simulink model "dds_cwg_32k_dual_test"  (R2016b) (continued)
== system dds_cwg_32k_dual_test/DDC == (continued)
      | pipeline_init(gcb,'latency',latency);
      settings: AttributesFormatString=Latency = %<latency>
      mask Display:
      | port_label('input', 1, 'd');
      | port_label('output', 1, 'q');
      | disp(['Z^-', num2str(latency)]);
  pipeline11 : Subsystem  Commented=on  AncestorBlock=casper_library_delays/pipeline  mask(pipeline){latency=2}
      mask help:
      | An explicitly laid-out delay line to avoid mapping to SRL16s
      | for use in pipelining to help achieve timing closure.
      mask init:
      | pipeline_init(gcb,'latency',latency);
      settings: AttributesFormatString=Latency = %<latency>
      mask Display:
      | port_label('input', 1, 'd');
      | port_label('output', 1, 'q');
      | disp(['Z^-', num2str(latency)]);
  pipeline12 : Subsystem  Commented=on  AncestorBlock=casper_library_delays/pipeline  mask(pipeline){latency=2}
      mask help:
      | An explicitly laid-out delay line to avoid mapping to SRL16s
      | for use in pipelining to help achieve timing closure.
      mask init:
      | pipeline_init(gcb,'latency',latency);
      settings: AttributesFormatString=Latency = %<latency>
      mask Display:
      | port_label('input', 1, 'd');
      | port_label('output', 1, 'q');
      | disp(['Z^-', num2str(latency)]);
  pipeline13 : Subsystem  Commented=on  AncestorBlock=casper_library_delays/pipeline  mask(pipeline){latency=2}
      mask help:
      | An explicitly laid-out delay line to avoid mapping to SRL16s
      | for use in pipelining to help achieve timing closure.
      mask init:
      | pipeline_init(gcb,'latency',latency);
      settings: AttributesFormatString=Latency = %<latency>
      mask Display:
      | port_label('input', 1, 'd');
      | port_label('output', 1, 'q');
      | disp(['Z^-', num2str(latency)]);
  pipeline14 : Subsystem  Commented=on  AncestorBlock=casper_library_delays/pipeline  mask(pipeline){latency=2}
      mask help:
      | An explicitly laid-out delay line to avoid mapping to SRL16s
      | for use in pipelining to help achieve timing closure.
      mask init:
      | pipeline_init(gcb,'latency',latency);
      settings: AttributesFormatString=Latency = %<latency>
      mask Display:
      | port_label('input', 1, 'd');
      | port_label('output', 1, 'q');
      | disp(['Z^-', num2str(latency)]);
  pipeline15 : Subsystem  Commented=on  AncestorBlock=casper_library_delays/pipeline  mask(pipeline){latency=2}
      mask help:
      | An explicitly laid-out delay line to avoid mapping to SRL16s
      | for use in pipelining to help achieve timing closure.
      mask init:
      | pipeline_init(gcb,'latency',latency);
      settings: AttributesFormatString=Latency = %<latency>
      mask Display:
      | port_label('input', 1, 'd');
      | port_label('output', 1, 'q');
      | disp(['Z^-', num2str(latency)]);
  pipeline16 : Subsystem  Commented=on  AncestorBlock=casper_library_delays/pipeline  mask(pipeline){latency=2}
      mask help:
      | An explicitly laid-out delay line to avoid mapping to SRL16s
      | for use in pipelining to help achieve timing closure.
      mask init:
      | pipeline_init(gcb,'latency',latency);
      settings: AttributesFormatString=Latency = %<latency>
      mask Display:
      | port_label('input', 1, 'd');
      | port_label('output', 1, 'q');
      | disp(['Z^-', num2str(latency)]);
  pipeline17 : Subsystem  Commented=on  AncestorBlock=casper_library_delays/pipeline  mask(pipeline){latency=2}
      mask help:
      | An explicitly laid-out delay line to avoid mapping to SRL16s
      | for use in pipelining to help achieve timing closure.
      mask init:
      | pipeline_init(gcb,'latency',latency);
      settings: AttributesFormatString=Latency = %<latency>
      mask Display:
      | port_label('input', 1, 'd');
      | port_label('output', 1, 'q');
      | disp(['Z^-', num2str(latency)]);
  pipeline18 : Subsystem  Commented=on  AncestorBlock=casper_library_delays/pipeline  mask(pipeline){latency=2}
      mask help:
      | An explicitly laid-out delay line to avoid mapping to SRL16s
      | for use in pipelining to help achieve timing closure.
      mask init:
      | pipeline_init(gcb,'latency',latency);
      settings: AttributesFormatString=Latency = %<latency>
      mask Display:
      | port_label('input', 1, 'd');
      | port_label('output', 1, 'q');
      | disp(['Z^-', num2str(latency)]);
  pipeline19 : Subsystem  Commented=on  AncestorBlock=casper_library_delays/pipeline  mask(pipeline){latency=2}
      mask help:
      | An explicitly laid-out delay line to avoid mapping to SRL16s
      | for use in pipelining to help achieve timing closure.
      mask init:
      | pipeline_init(gcb,'latency',latency);
      settings: AttributesFormatString=Latency = %<latency>
      mask Display:
      | port_label('input', 1, 'd');
      | port_label('output', 1, 'q');
      | disp(['Z^-', num2str(latency)]);
  pipeline2 : Subsystem  Commented=on  AncestorBlock=casper_library_delays/pipeline  mask(pipeline){latency=1}
      mask help:
      | An explicitly laid-out delay line to avoid mapping to SRL16s
      | for use in pipelining to help achieve timing closure.
      mask init:
      | pipeline_init(gcb,'latency',latency);
      settings: AttributesFormatString=Latency = %<latency>
      mask Display:
      | port_label('input', 1, 'd');
      | port_label('output', 1, 'q');
      | disp(['Z^-', num2str(latency)]);
  pipeline20 : Subsystem  Commented=on  AncestorBlock=casper_library_delays/pipeline  mask(pipeline){latency=2}
      mask help:
      | An explicitly laid-out delay line to avoid mapping to SRL16s
      | for use in pipelining to help achieve timing closure.
      mask init:
      | pipeline_init(gcb,'latency',latency);
      settings: AttributesFormatString=Latency = %<latency>
      mask Display:
      | port_label('input', 1, 'd');
      | port_label('output', 1, 'q');
      | disp(['Z^-', num2str(latency)]);
  pipeline21 : Subsystem  AncestorBlock=casper_library_delays/pipeline  mask(pipeline){latency=2}
      mask help:
      | An explicitly laid-out delay line to avoid mapping to SRL16s
      | for use in pipelining to help achieve timing closure.
      mask init:
      | pipeline_init(gcb,'latency',latency);
      settings: AttributesFormatString=Latency = %<latency>
      mask Display:
      | port_label('input', 1, 'd');
      | port_label('output', 1, 'q');
      | disp(['Z^-', num2str(latency)]);
  pipeline22 : Subsystem  AncestorBlock=casper_library_delays/pipeline  mask(pipeline){latency=2}
      mask help:
      | An explicitly laid-out delay line to avoid mapping to SRL16s
      | for use in pipelining to help achieve timing closure.
      mask init:
      | pipeline_init(gcb,'latency',latency);
      settings: AttributesFormatString=Latency = %<latency>
      mask Display:
      | port_label('input', 1, 'd');
      | port_label('output', 1, 'q');
      | disp(['Z^-', num2str(latency)]);
  pipeline23 : Subsystem  Commented=on  AncestorBlock=casper_library_delays/pipeline  mask(pipeline){latency=2}
      mask help:
      | An explicitly laid-out delay line to avoid mapping to SRL16s
      | for use in pipelining to help achieve timing closure.
      mask init:
      | pipeline_init(gcb,'latency',latency);
      settings: AttributesFormatString=Latency = %<latency>
      mask Display:
      | port_label('input', 1, 'd');
      | port_label('output', 1, 'q');
      | disp(['Z^-', num2str(latency)]);
  pipeline24 : Subsystem  Commented=on  AncestorBlock=casper_library_delays/pipeline  mask(pipeline){latency=2}
      mask help:
      | An explicitly laid-out delay line to avoid mapping to SRL16s
      | for use in pipelining to help achieve timing closure.
      mask init:
      | pipeline_init(gcb,'latency',latency);
      settings: AttributesFormatString=Latency = %<latency>
      mask Display:
      | port_label('input', 1, 'd');
      | port_label('output', 1, 'q');
      | disp(['Z^-', num2str(latency)]);
  pipeline25 : Subsystem  Commented=on  AncestorBlock=casper_library_delays/pipeline  mask(pipeline){latency=1}
      mask help:
      | An explicitly laid-out delay line to avoid mapping to SRL16s
      | for use in pipelining to help achieve timing closure.
      mask init:
      | pipeline_init(gcb,'latency',latency);
      settings: AttributesFormatString=Latency = %<latency>
      mask Display:
      | port_label('input', 1, 'd');
      | port_label('output', 1, 'q');
      | disp(['Z^-', num2str(latency)]);
  pipeline26 : Subsystem  Commented=on  AncestorBlock=casper_library_delays/pipeline  mask(pipeline){latency=2}
      mask help:
      | An explicitly laid-out delay line to avoid mapping to SRL16s
      | for use in pipelining to help achieve timing closure.
      mask init:
      | pipeline_init(gcb,'latency',latency);
      settings: AttributesFormatString=Latency = %<latency>
      mask Display:
      | port_label('input', 1, 'd');
      | port_label('output', 1, 'q');
      | disp(['Z^-', num2str(latency)]);
  pipeline27 : Subsystem  Commented=on  AncestorBlock=casper_library_delays/pipeline  mask(pipeline){latency=2}
      mask help:
      | An explicitly laid-out delay line to avoid mapping to SRL16s
      | for use in pipelining to help achieve timing closure.
      mask init:
      | pipeline_init(gcb,'latency',latency);
      settings: AttributesFormatString=Latency = %<latency>
      mask Display:
      | port_label('input', 1, 'd');
      | port_label('output', 1, 'q');
      | disp(['Z^-', num2str(latency)]);
  pipeline28 : Subsystem  Commented=on  AncestorBlock=casper_library_delays/pipeline  mask(pipeline){latency=2}
      mask help:
      | An explicitly laid-out delay line to avoid mapping to SRL16s
      | for use in pipelining to help achieve timing closure.
      mask init:
      | pipeline_init(gcb,'latency',latency);
      settings: AttributesFormatString=Latency = %<latency>
      mask Display:
      | port_label('input', 1, 'd');
      | port_label('output', 1, 'q');
      | disp(['Z^-', num2str(latency)]);
  pipeline29 : Subsystem  Commented=on  AncestorBlock=casper_library_delays/pipeline  mask(pipeline){latency=2}
      mask help:
      | An explicitly laid-out delay line to avoid mapping to SRL16s
      | for use in pipelining to help achieve timing closure.
      mask init:
      | pipeline_init(gcb,'latency',latency);
      settings: AttributesFormatString=Latency = %<latency>
      mask Display:
      | port_label('input', 1, 'd');
      | port_label('output', 1, 'q');
      | disp(['Z^-', num2str(latency)]);
  pipeline3 : Subsystem  Commented=on  AncestorBlock=casper_library_delays/pipeline  mask(pipeline){latency=2}
      mask help:
      | An explicitly laid-out delay line to avoid mapping to SRL16s
      | for use in pipelining to help achieve timing closure.
      mask init:
      | pipeline_init(gcb,'latency',latency);
      settings: AttributesFormatString=Latency = %<latency>
      mask Display:
      | port_label('input', 1, 'd');
      | port_label('output', 1, 'q');
      | disp(['Z^-', num2str(latency)]);
  pipeline30 : Subsystem  Commented=on  AncestorBlock=casper_library_delays/pipeline  mask(pipeline){latency=2}
      mask help:
      | An explicitly laid-out delay line to avoid mapping to SRL16s
      | for use in pipelining to help achieve timing closure.
      mask init:
      | pipeline_init(gcb,'latency',latency);
      settings: AttributesFormatString=Latency = %<latency>
      mask Display:
      | port_label('input', 1, 'd');
      | port_label('output', 1, 'q');
      | disp(['Z^-', num2str(latency)]);
  pipeline31 : Subsystem  Commented=on  AncestorBlock=casper_library_delays/pipeline  mask(pipeline){latency=2}
      mask help:
      | An explicitly laid-out delay line to avoid mapping to SRL16s
      | for use in pipelining to help achieve timing closure.
      mask init:
      | pipeline_init(gcb,'latency',latency);
      settings: AttributesFormatString=Latency = %<latency>
      mask Display:
      | port_label('input', 1, 'd');
      | port_label('output', 1, 'q');
      | disp(['Z^-', num2str(latency)]);
  pipeline32 : Subsystem  Commented=on  AncestorBlock=casper_library_delays/pipeline  mask(pipeline){latency=1}
      mask help:
      | An explicitly laid-out delay line to avoid mapping to SRL16s
      | for use in pipelining to help achieve timing closure.
      mask init:
      | pipeline_init(gcb,'latency',latency);
      settings: AttributesFormatString=Latency = %<latency>
      mask Display:
      | port_label('input', 1, 'd');
      | port_label('output', 1, 'q');
      | disp(['Z^-', num2str(latency)]);
  pipeline33 : Subsystem  Commented=on  AncestorBlock=casper_library_delays/pipeline  mask(pipeline){latency=2}
      mask help:
      | An explicitly laid-out delay line to avoid mapping to SRL16s
      | for use in pipelining to help achieve timing closure.
      mask init:
      | pipeline_init(gcb,'latency',latency);
      settings: AttributesFormatString=Latency = %<latency>
      mask Display:
      | port_label('input', 1, 'd');
      | port_label('output', 1, 'q');
      | disp(['Z^-', num2str(latency)]);
  pipeline34 : Subsystem  Commented=on  AncestorBlock=casper_library_delays/pipeline  mask(pipeline){latency=2}
      mask help:
      | An explicitly laid-out delay line to avoid mapping to SRL16s
      | for use in pipelining to help achieve timing closure.
      mask init:
      | pipeline_init(gcb,'latency',latency);
      settings: AttributesFormatString=Latency = %<latency>
      mask Display:
      | port_label('input', 1, 'd');
      | port_label('output', 1, 'q');
      | disp(['Z^-', num2str(latency)]);
  pipeline35 : Subsystem  Commented=on  AncestorBlock=casper_library_delays/pipeline  mask(pipeline){latency=2}
      mask help:
      | An explicitly laid-out delay line to avoid mapping to SRL16s
      | for use in pipelining to help achieve timing closure.
      mask init:
      | pipeline_init(gcb,'latency',latency);
      settings: AttributesFormatString=Latency = %<latency>
      mask Display:
      | port_label('input', 1, 'd');
      | port_label('output', 1, 'q');
      | disp(['Z^-', num2str(latency)]);
  pipeline36 : Subsystem  Commented=on  AncestorBlock=casper_library_delays/pipeline  mask(pipeline){latency=2}
      mask help:
      | An explicitly laid-out delay line to avoid mapping to SRL16s
      | for use in pipelining to help achieve timing closure.
      mask init:
      | pipeline_init(gcb,'latency',latency);
      settings: AttributesFormatString=Latency = %<latency>
      mask Display:
      | port_label('input', 1, 'd');
      | port_label('output', 1, 'q');
      | disp(['Z^-', num2str(latency)]);
  pipeline37 : Subsystem  Commented=on  AncestorBlock=casper_library_delays/pipeline  mask(pipeline){latency=2}
      mask help:
      | An explicitly laid-out delay line to avoid mapping to SRL16s
      | for use in pipelining to help achieve timing closure.
      mask init:
      | pipeline_init(gcb,'latency',latency);
      settings: AttributesFormatString=Latency = %<latency>
      mask Display:
      | port_label('input', 1, 'd');
      | port_label('output', 1, 'q');
      | disp(['Z^-', num2str(latency)]);
  pipeline38 : Subsystem  Commented=on  AncestorBlock=casper_library_delays/pipeline  mask(pipeline){latency=2}
      mask help:
      | An explicitly laid-out delay line to avoid mapping to SRL16s
      | for use in pipelining to help achieve timing closure.
      mask init:
      | pipeline_init(gcb,'latency',latency);
      settings: AttributesFormatString=Latency = %<latency>
      mask Display:
      | port_label('input', 1, 'd');
      | port_label('output', 1, 'q');
      | disp(['Z^-', num2str(latency)]);
  pipeline39 : Subsystem  AncestorBlock=casper_library_delays/pipeline  mask(pipeline){latency=2}
      mask help:
      | An explicitly laid-out delay line to avoid mapping to SRL16s
      | for use in pipelining to help achieve timing closure.
      mask init:
      | pipeline_init(gcb,'latency',latency);
      settings: AttributesFormatString=Latency = %<latency>
      mask Display:
      | port_label('input', 1, 'd');
      | port_label('output', 1, 'q');
      | disp(['Z^-', num2str(latency)]);
  pipeline4 : Subsystem  Commented=on  AncestorBlock=casper_library_delays/pipeline  mask(pipeline){latency=2}
      mask help:
      | An explicitly laid-out delay line to avoid mapping to SRL16s
      | for use in pipelining to help achieve timing closure.
      mask init:
      | pipeline_init(gcb,'latency',latency);
      settings: AttributesFormatString=Latency = %<latency>
      mask Display:
      | port_label('input', 1, 'd');
      | port_label('output', 1, 'q');
      | disp(['Z^-', num2str(latency)]);
  pipeline40 : Subsystem  AncestorBlock=casper_library_delays/pipeline  mask(pipeline){latency=2}
      mask help:
      | An explicitly laid-out delay line to avoid mapping to SRL16s
      | for use in pipelining to help achieve timing closure.
      mask init:
      | pipeline_init(gcb,'latency',latency);
      settings: AttributesFormatString=Latency = %<latency>
      mask Display:
      | port_label('input', 1, 'd');
      | port_label('output', 1, 'q');
      | disp(['Z^-', num2str(latency)]);
  pipeline41 : Subsystem  Commented=on  AncestorBlock=casper_library_delays/pipeline  mask(pipeline){latency=2}
      mask help:
      | An explicitly laid-out delay line to avoid mapping to SRL16s
      | for use in pipelining to help achieve timing closure.
      mask init:
      | pipeline_init(gcb,'latency',latency);
      settings: AttributesFormatString=Latency = %<latency>
      mask Display:
      | port_label('input', 1, 'd');
      | port_label('output', 1, 'q');
      | disp(['Z^-', num2str(latency)]);
  pipeline42 : Subsystem  Commented=on  AncestorBlock=casper_library_delays/pipeline  mask(pipeline){latency=2}
      mask help:
      | An explicitly laid-out delay line to avoid mapping to SRL16s
      | for use in pipelining to help achieve timing closure.
      mask init:
      | pipeline_init(gcb,'latency',latency);
      settings: AttributesFormatString=Latency = %<latency>
      mask Display:
      | port_label('input', 1, 'd');
      | port_label('output', 1, 'q');
      | disp(['Z^-', num2str(latency)]);
  pipeline43 : Subsystem  Commented=on  AncestorBlock=casper_library_delays/pipeline  mask(pipeline){latency=2}
      mask help:
      | An explicitly laid-out delay line to avoid mapping to SRL16s
      | for use in pipelining to help achieve timing closure.
      mask init:
      | pipeline_init(gcb,'latency',latency);
      settings: AttributesFormatString=Latency = %<latency>
      mask Display:
      | port_label('input', 1, 'd');
      | port_label('output', 1, 'q');
      | disp(['Z^-', num2str(latency)]);
  pipeline44 : Subsystem  Commented=on  AncestorBlock=casper_library_delays/pipeline  mask(pipeline){latency=1}
      mask help:
      | An explicitly laid-out delay line to avoid mapping to SRL16s
      | for use in pipelining to help achieve timing closure.
      mask init:
      | pipeline_init(gcb,'latency',latency);
      settings: AttributesFormatString=Latency = %<latency>
      mask Display:
      | port_label('input', 1, 'd');
      | port_label('output', 1, 'q');
      | disp(['Z^-', num2str(latency)]);
  pipeline5 : Subsystem  Commented=on  AncestorBlock=casper_library_delays/pipeline  mask(pipeline){latency=2}
      mask help:
      | An explicitly laid-out delay line to avoid mapping to SRL16s
      | for use in pipelining to help achieve timing closure.
      mask init:
      | pipeline_init(gcb,'latency',latency);
      settings: AttributesFormatString=Latency = %<latency>
      mask Display:
      | port_label('input', 1, 'd');
      | port_label('output', 1, 'q');
      | disp(['Z^-', num2str(latency)]);
  pipeline6 : Subsystem  Commented=on  AncestorBlock=casper_library_delays/pipeline  mask(pipeline){latency=2}
      mask help:
      | An explicitly laid-out delay line to avoid mapping to SRL16s
      | for use in pipelining to help achieve timing closure.
      mask init:
      | pipeline_init(gcb,'latency',latency);
      settings: AttributesFormatString=Latency = %<latency>
      mask Display:
      | port_label('input', 1, 'd');
      | port_label('output', 1, 'q');
      | disp(['Z^-', num2str(latency)]);
  pipeline7 : Subsystem  Commented=on  AncestorBlock=casper_library_delays/pipeline  mask(pipeline){latency=2}
      mask help:
      | An explicitly laid-out delay line to avoid mapping to SRL16s
      | for use in pipelining to help achieve timing closure.
      mask init:
      | pipeline_init(gcb,'latency',latency);
      settings: AttributesFormatString=Latency = %<latency>
      mask Display:
      | port_label('input', 1, 'd');
      | port_label('output', 1, 'q');
      | disp(['Z^-', num2str(latency)]);
  pipeline8 : Subsystem  Commented=on  AncestorBlock=casper_library_delays/pipeline  mask(pipeline){latency=2}
      mask help:
      | An explicitly laid-out delay line to avoid mapping to SRL16s
      | for use in pipelining to help achieve timing closure.
      mask init:
      | pipeline_init(gcb,'latency',latency);
      settings: AttributesFormatString=Latency = %<latency>
      mask Display:
      | port_label('input', 1, 'd');
      | port_label('output', 1, 'q');
      | disp(['Z^-', num2str(latency)]);
  pipeline9 : Subsystem  Commented=on  AncestorBlock=casper_library_delays/pipeline  mask(pipeline){latency=2}
      mask help:
      | An explicitly laid-out delay line to avoid mapping to SRL16s
      | for use in pipelining to help achieve timing closure.
      mask init:
      | pipeline_init(gcb,'latency',latency);
      settings: AttributesFormatString=Latency = %<latency>
      mask Display:
      | port_label('input', 1, 'd');
      | port_label('output', 1, 'q');
      | disp(['Z^-', num2str(latency)]);
  ri_to_c1 : casper_library_misc/ri_to_c  Commented=on
  snap_ddc : Subsystem  Tag=casper:bitsnap  Commented=on  AncestorBlock=casper_library_scopes/bitfield_snapshot  mask(bitsnap){io_names=[fir_re fir_re_u fir_im dv sync], io_widths=[18 18 18 1 1], io_bps=[17 0 17 0 0], io_types=[1 0 1 2 2], show_format=on, snap_storage=bram, snap_dram_dimm=2, snap_dram_clock=250, snap_nsamples=10, snap_data_width=64, snap_offset=on, snap_circap=off, snap_value=off, snap_ext_arm=off, snap_ext_circ=off, snap_provide_outputs=on, snap_use_dsp48=on, snap_delay=0, extra_names=[c d], extra_widths=[30 2], extra_bps=[0 0], extra_types=0}
      mask prompts: io_names "Names [msb...lsb]"; io_widths "Bitwidths [msb...lsb]"; io_bps "Binary pts [msb...lsb]"; io_types "Data types [msb...lsb], ufix=0, fix=1, bool=2"; show_format "Print format string?"; snap_storage "Storage medium"; snap_dram_dimm "DRAM dimm"; snap_dram_clock "DRAM clock rate"; snap_nsamples "Number of Samples (2^?)"; snap_data_width "Data width"; snap_offset "Start delay support"; snap_circap "Circular capture support"; snap_value "Extra value capture support"; snap_ext_arm "External arm support"; snap_ext_circ "External circular capture support"; snap_provide_outputs "Provide outputs"; snap_use_dsp48 "Use DSP48s to implement counters"; snap_delay "Pre-snapshot delay"; extra_names "Names [msb...lsb]"; extra_widths "Widths [msb...lsb]"; extra_bps "Binary pts [msb...lsb]"; extra_types "Data types [msb...lsb], ufix=0, fix=1, bool=2"
      mask help:
      | A wrapped snapshot block. Allows specification of inputs to a snapblock word so the toolflow can automatically set up the scope.
      | See documentation for snapshot for explanation of parameters.
      mask init:
      | bitsnap_init(gcb);
      settings: AttributesFormatString=|
      | snap(5):f18.17,uf18.0,f18.17,b,b = 56 bits\n64 wide, 1024 deep
      | debugID: 41605630
      mask TypeOptions snap_storage: bram | dram
      mask Callback snap_storage: bitsnap_callback();
      mask TypeOptions snap_dram_dimm: 1 | 2 | 3 | 4
      mask TypeOptions snap_dram_clock: 200 | 250 | 266 | 300 | 333
      mask TypeOptions snap_data_width: 8 | 16 | 32 | 64 | 128
      mask Callback snap_value: bitsnap_callback();
      mask Callback snap_ext_arm: bitsnap_callback();
      mask Callback snap_ext_circ: bitsnap_callback();
      mask Callback snap_provide_outputs: bitsnap_callback();
  snap_ddc_dgb : Subsystem  Tag=casper:bitsnap  Commented=on  AncestorBlock=casper_library_scopes/bitfield_snapshot  mask(bitsnap){io_names=[dec_r1 dec_i1 dec_r9 dec_i9 sync_in dv_in ddc_rout ddc_iout sync_out dv_out], io_widths=[18 18 18 18 1 1 18 18 1 1], io_bps=[17 17 17 17 0 0 17 17 0 0], io_types=[1 1 1 1 2 2 1 1 2 2], show_format=on, snap_storage=bram, snap_dram_dimm=2, snap_dram_clock=250, snap_nsamples=10, snap_data_width=128, snap_offset=on, snap_circap=off, snap_value=off, snap_ext_arm=off, snap_ext_circ=off, snap_provide_outputs=on, snap_use_dsp48=on, snap_delay=0, extra_names=[c d], extra_widths=[30 2], extra_bps=[0 0], extra_types=0}
      mask prompts: io_names "Names [msb...lsb]"; io_widths "Bitwidths [msb...lsb]"; io_bps "Binary pts [msb...lsb]"; io_types "Data types [msb...lsb], ufix=0, fix=1, bool=2"; show_format "Print format string?"; snap_storage "Storage medium"; snap_dram_dimm "DRAM dimm"; snap_dram_clock "DRAM clock rate"; snap_nsamples "Number of Samples (2^?)"; snap_data_width "Data width"; snap_offset "Start delay support"; snap_circap "Circular capture support"; snap_value "Extra value capture support"; snap_ext_arm "External arm support"; snap_ext_circ "External circular capture support"; snap_provide_outputs "Provide outputs"; snap_use_dsp48 "Use DSP48s to implement counters"; snap_delay "Pre-snapshot delay"; extra_names "Names [msb...lsb]"; extra_widths "Widths [msb...lsb]"; extra_bps "Binary pts [msb...lsb]"; extra_types "Data types [msb...lsb], ufix=0, fix=1, bool=2"
      mask help:
      | A wrapped snapshot block. Allows specification of inputs to a snapblock word so the toolflow can automatically set up the scope.
      | See documentation for snapshot for explanation of parameters.
      mask init:
      | bitsnap_init(gcb);
      settings: AttributesFormatString=|
      | snap(10):f18.17,f18.17,f18.17,f18.17,b,b,f18.17,f18.17,b,b = 112 bits\n128 wide, 1024 deep
      | debugID: 108545790
      mask TypeOptions snap_storage: bram | dram
      mask Callback snap_storage: bitsnap_callback();
      mask TypeOptions snap_dram_dimm: 1 | 2 | 3 | 4
      mask TypeOptions snap_dram_clock: 200 | 250 | 266 | 300 | 333
      mask TypeOptions snap_data_width: 8 | 16 | 32 | 64 | 128
      mask Callback snap_value: bitsnap_callback();
      mask Callback snap_ext_arm: bitsnap_callback();
      mask Callback snap_ext_circ: bitsnap_callback();
      mask Callback snap_provide_outputs: bitsnap_callback();
  snap_mix : Subsystem  Tag=casper:bitsnap  Commented=on  AncestorBlock=casper_library_scopes/bitfield_snapshot  mask(bitsnap){io_names=[mix0 mix7 sync dv], io_widths=[18 18 1 1], io_bps=[17 0 0 0], io_types=[1 0 2 2], show_format=on, snap_storage=bram, snap_dram_dimm=2, snap_dram_clock=250, snap_nsamples=10, snap_data_width=64, snap_offset=on, snap_circap=off, snap_value=off, snap_ext_arm=off, snap_ext_circ=off, snap_provide_outputs=on, snap_use_dsp48=on, snap_delay=0, extra_names=[c d], extra_widths=[30 2], extra_bps=[0 0], extra_types=0}
      mask prompts: io_names "Names [msb...lsb]"; io_widths "Bitwidths [msb...lsb]"; io_bps "Binary pts [msb...lsb]"; io_types "Data types [msb...lsb], ufix=0, fix=1, bool=2"; show_format "Print format string?"; snap_storage "Storage medium"; snap_dram_dimm "DRAM dimm"; snap_dram_clock "DRAM clock rate"; snap_nsamples "Number of Samples (2^?)"; snap_data_width "Data width"; snap_offset "Start delay support"; snap_circap "Circular capture support"; snap_value "Extra value capture support"; snap_ext_arm "External arm support"; snap_ext_circ "External circular capture support"; snap_provide_outputs "Provide outputs"; snap_use_dsp48 "Use DSP48s to implement counters"; snap_delay "Pre-snapshot delay"; extra_names "Names [msb...lsb]"; extra_widths "Widths [msb...lsb]"; extra_bps "Binary pts [msb...lsb]"; extra_types "Data types [msb...lsb], ufix=0, fix=1, bool=2"
      mask help:
      | A wrapped snapshot block. Allows specification of inputs to a snapblock word so the toolflow can automatically set up the scope.
      | See documentation for snapshot for explanation of parameters.
      mask init:
      | bitsnap_init(gcb);
      settings: AttributesFormatString=|
      | snap(4):f18.17,uf18.0,b,b = 38 bits\n64 wide, 1024 deep
      | debugID: 36075749
      mask TypeOptions snap_storage: bram | dram
      mask Callback snap_storage: bitsnap_callback();
      mask TypeOptions snap_dram_dimm: 1 | 2 | 3 | 4
      mask TypeOptions snap_dram_clock: 200 | 250 | 266 | 300 | 333
      mask TypeOptions snap_data_width: 8 | 16 | 32 | 64 | 128
      mask Callback snap_value: bitsnap_callback();
      mask Callback snap_ext_arm: bitsnap_callback();
      mask Callback snap_ext_circ: bitsnap_callback();
      mask Callback snap_provide_outputs: bitsnap_callback();
  snap_mult : Subsystem  Tag=casper:bitsnap  Commented=on  AncestorBlock=casper_library_scopes/bitfield_snapshot  mask(bitsnap){io_names=[ain0 ain7 bin0 bin7 mout0 mix0 mix7 sync_in dv_in mix_sync mix_dv dec_sync dec_dv], io_widths=[10 10 18 18 18 18 18 1 1 1 1 1 1], io_bps=[9 0 17 0 17 17 0 0 0 0 0 0 0], io_types=[1 0 1 0 1 1 0 2 2 2 2 2 2], show_format=on, snap_storage=bram, snap_dram_dimm=2, snap_dram_clock=250, snap_nsamples=10, snap_data_width=128, snap_offset=on, snap_circap=off, snap_value=off, snap_ext_arm=off, snap_ext_circ=off, snap_provide_outputs=on, snap_use_dsp48=on, snap_delay=0, extra_names=[c d], extra_widths=[30 2], extra_bps=[0 0], extra_types=0}
      mask prompts: io_names "Names [msb...lsb]"; io_widths "Bitwidths [msb...lsb]"; io_bps "Binary pts [msb...lsb]"; io_types "Data types [msb...lsb], ufix=0, fix=1, bool=2"; show_format "Print format string?"; snap_storage "Storage medium"; snap_dram_dimm "DRAM dimm"; snap_dram_clock "DRAM clock rate"; snap_nsamples "Number of Samples (2^?)"; snap_data_width "Data width"; snap_offset "Start delay support"; snap_circap "Circular capture support"; snap_value "Extra value capture support"; snap_ext_arm "External arm support"; snap_ext_circ "External circular capture support"; snap_provide_outputs "Provide outputs"; snap_use_dsp48 "Use DSP48s to implement counters"; snap_delay "Pre-snapshot delay"; extra_names "Names [msb...lsb]"; extra_widths "Widths [msb...lsb]"; extra_bps "Binary pts [msb...lsb]"; extra_types "Data types [msb...lsb], ufix=0, fix=1, bool=2"
      mask help:
      | A wrapped snapshot block. Allows specification of inputs to a snapblock word so the toolflow can automatically set up the scope.
      | See documentation for snapshot for explanation of parameters.
      mask init:
      | bitsnap_init(gcb);
      settings: AttributesFormatString=|
      | snap(13):f10.9,uf10.0,f18.17,uf18.0,f18.17,f18.17,uf18.0,b,b,b,b,b,b = 116 bits\n128 wide, 1024 deep
      | debugID: 110628845
      mask TypeOptions snap_storage: bram | dram
      mask Callback snap_storage: bitsnap_callback();
      mask TypeOptions snap_dram_dimm: 1 | 2 | 3 | 4
      mask TypeOptions snap_dram_clock: 200 | 250 | 266 | 300 | 333
      mask TypeOptions snap_data_width: 8 | 16 | 32 | 64 | 128
      mask Callback snap_value: bitsnap_callback();
      mask Callback snap_ext_arm: bitsnap_callback();
      mask Callback snap_ext_circ: bitsnap_callback();
      mask Callback snap_provide_outputs: bitsnap_callback();
  ddc_sync : Outport
  ddc_pol0 : Outport  Port=2
  ddc_pol1 : Outport  Port=3
  ddc_dv : Outport  Port=4
  ddc_of : Outport  Port=5
  tag scope: dec_d80_0=global, dec_d80_1=global, dec_dv=global, dec_sync=global, mix_80_0=global, mix_dvalid=global, mix_sync=global
  wires:
  bus_mult/2(misco) -> bus_expand/1(bus_in)
  [ddc_sync] -> ddc_sync/1
  [ddc_pol0] -> ddc_pol0/1
  [ddc_pol1] -> ddc_pol1/1
  pol1_data/1 -> bus_mult1/1(a)
  osc1/1 -> bus_mult1/2(b)
  [cnt_rst] -> pipeline22/1(d)
  Counter1/1 -> ddc_out_sync_cnt/1(out_reg)
  pipeline21/1(q) -> Counter1/2
  pipeline22/1(q) -> Counter1/1
  [ddc_sync] -> pipeline21/1(d)
  [ddc_dvalid] -> ddc_dv/1
  Delay3/1 -> bus_expand5/1(bus_in)
  Delay5/1 -> bus_expand9/1(bus_in)
  bus_expand9/1(msb_out16) -> dec_fir_async1/3(real1)
  bus_expand9/2(out15) -> dec_fir_async1/4(imag1)
  bus_expand9/3(out14) -> dec_fir_async1/5(real2)
  bus_expand9/4(out13) -> dec_fir_async1/6(imag2)
  bus_expand9/5(out12) -> dec_fir_async1/7(real3)
  bus_expand9/6(out11) -> dec_fir_async1/8(imag3)
  bus_expand9/7(out10) -> dec_fir_async1/9(real4)
  bus_expand9/8(out9) -> dec_fir_async1/10(imag4)
  bus_expand9/9(out8) -> dec_fir_async1/11(real5)
  bus_expand9/10(out7) -> dec_fir_async1/12(imag5)
  bus_expand9/11(out6) -> dec_fir_async1/13(real6)
  bus_expand9/12(out5) -> dec_fir_async1/14(imag6)
  bus_expand9/13(out4) -> dec_fir_async1/15(real7)
  bus_expand9/14(out3) -> dec_fir_async1/16(imag7)
  bus_expand9/15(out2) -> dec_fir_async1/17(real8)
  bus_expand9/16(lsb_out1) -> dec_fir_async1/18(imag8)
  bus_expand10/1(msb_out16) -> dec_fir_async1/19(real9)
  bus_expand10/3(out14) -> dec_fir_async1/21(real10)
  bus_expand10/2(out15) -> dec_fir_async1/20(imag9)
  bus_expand10/4(out13) -> dec_fir_async1/22(imag10)
  bus_expand10/5(out12) -> dec_fir_async1/23(real11)
  bus_expand10/7(out10) -> dec_fir_async1/25(real12)
  bus_expand10/6(out11) -> dec_fir_async1/24(imag11)
  bus_expand10/8(out9) -> dec_fir_async1/26(imag12)
  bus_expand10/9(out8) -> dec_fir_async1/27(real13)
  bus_expand10/10(out7) -> dec_fir_async1/28(imag13)
  bus_expand10/11(out6) -> dec_fir_async1/29(real14)
  bus_expand10/12(out5) -> dec_fir_async1/30(imag14)
  bus_expand10/14(out3) -> dec_fir_async1/32(imag15)
  bus_expand10/13(out4) -> dec_fir_async1/31(real15)
  bus_expand10/15(out2) -> dec_fir_async1/33(real16)
  bus_expand10/16(lsb_out1) -> dec_fir_async1/34(imag16)
  dec_fir_async1/2(dout) -> ?
  [cnt_rst] -> pipeline40/1(d)
  Counter3/1 -> ddc_in_sync_cnt/1(out_reg)
  pipeline39/1(q) -> Counter3/2
  pipeline40/1(q) -> Counter3/1
  [dec_sync] -> pipeline39/1(d)
  [dec_dv] -> dec_fir_async1/2(dv_in)
  [dec_sync] -> dec_fir_async1/1(sync_in)
  [dec_d80_1] -> Delay5/1, bus_expand10/1(bus_in)
  [dec_d80_0] -> bus_expand6/1(bus_in), Delay3/1
  [ddc_of_pol0] -> Concat1/1
  [ddc_of_pol1] -> Concat1/2
  Concat1/1 -> ddc_of/1
  Constant/1 -> [ddc_of_pol1], [ddc_of_pol0]
  bus_expand6/16(lsb_out1) -> dec_fir_async0/34(imag16)
  bus_expand6/15(out2) -> dec_fir_async0/33(real16)
  bus_expand6/14(out3) -> dec_fir_async0/32(imag15)
  bus_expand6/13(out4) -> dec_fir_async0/31(real15)
  bus_expand6/12(out5) -> dec_fir_async0/30(imag14)
  bus_expand6/11(out6) -> dec_fir_async0/29(real14)
  bus_expand6/10(out7) -> dec_fir_async0/28(imag13)
  bus_expand6/9(out8) -> dec_fir_async0/27(real13)
  bus_expand6/8(out9) -> dec_fir_async0/26(imag12)
  bus_expand6/7(out10) -> dec_fir_async0/25(real12)
  bus_expand6/6(out11) -> dec_fir_async0/24(imag11)
  bus_expand6/5(out12) -> dec_fir_async0/23(real11)
  bus_expand6/4(out13) -> dec_fir_async0/22(imag10)
  bus_expand6/3(out14) -> dec_fir_async0/21(real10)
  bus_expand6/2(out15) -> pipeline23/1(d), dec_fir_async0/20(imag9)
  bus_expand6/1(msb_out16) -> pipeline20/1(d), Gateway Out4/1, dec_fir_async0/19(real9)
  bus_expand5/16(lsb_out1) -> dec_fir_async0/18(imag8)
  bus_expand5/15(out2) -> dec_fir_async0/17(real8)
  bus_expand5/14(out3) -> dec_fir_async0/16(imag7)
  bus_expand5/13(out4) -> dec_fir_async0/15(real7)
  bus_expand5/12(out5) -> dec_fir_async0/14(imag6)
  bus_expand5/11(out6) -> dec_fir_async0/13(real6)
  bus_expand5/10(out7) -> dec_fir_async0/12(imag5)
  bus_expand5/9(out8) -> dec_fir_async0/11(real5)
  bus_expand5/8(out9) -> dec_fir_async0/10(imag4)
  bus_expand5/7(out10) -> dec_fir_async0/9(real4)
  bus_expand5/6(out11) -> dec_fir_async0/8(imag3)
  bus_expand5/5(out12) -> dec_fir_async0/7(real3)
  bus_expand5/4(out13) -> dec_fir_async0/6(imag2)
  bus_expand5/3(out14) -> dec_fir_async0/5(real2)
  bus_expand5/2(out15) -> pipeline19/1(d), dec_fir_async0/4(imag1)
  bus_expand5/1(msb_out16) -> pipeline18/1(d), Gateway Out3/1, dec_fir_async0/3(real1)
  convert_of1/1(dout) -> ri_to_c1/2
  convert_of/1(dout) -> ri_to_c1/1
  convert_of/2(of) -> Logical/1
  convert_of1/2(of) -> Logical/2
  From3/1 -> pipeline9/1(d), pipeline7/1(d)
  pipeline7/1(q) -> snap_ddc/4(in_dv)
  pipeline8/1(q) -> snap_ddc/5(in_sync)
  pipeline9/1(q) -> snap_ddc/6(we)
  pipeline25/1(q) -> snap_ddc/7(trig)
  From4/1 -> pipeline25/1(d), pipeline8/1(d)
  bus_expand12/2(lsb_out1) -> pipeline6/1(d)
  bus_expand12/1(msb_out2) -> pipeline1/1(d), pipeline10/1(d)
  From2/1 -> bus_expand12/1(bus_in)
  pipeline6/1(q) -> snap_ddc/3(in_fir_im)
  pipeline10/1(q) -> snap_ddc/1(in_fir_re)
  bus_expand4/2(lsb_out1) -> pipeline28/1(d)
  bus_expand4/1(msb_out2) -> pipeline27/1(d)
  pipeline18/1(q) -> snap_ddc_dgb/1(in_dec_r1)
  pipeline19/1(q) -> snap_ddc_dgb/2(in_dec_i1)
  pipeline20/1(q) -> snap_ddc_dgb/3(in_dec_r9)
  pipeline23/1(q) -> snap_ddc_dgb/4(in_dec_i9)
  pipeline24/1(q) -> snap_ddc_dgb/5(in_sync_in)
  pipeline26/1(q) -> snap_ddc_dgb/6(in_dv_in)
  pipeline27/1(q) -> snap_ddc_dgb/7(in_ddc_rout)
  pipeline28/1(q) -> snap_ddc_dgb/8(in_ddc_iout)
  pipeline29/1(q) -> snap_ddc_dgb/9(in_sync_out)
  pipeline30/1(q) -> snap_ddc_dgb/10(in_dv_out)
  pipeline31/1(q) -> snap_ddc_dgb/11(we)
  pipeline32/1(q) -> snap_ddc_dgb/12(trig)
  Register1/1 -> Logical1/1, Inverter/1
  edge_detect3/1(out) -> [dec_dv]
  edge_detect2/1(out) -> [dec_sync]
  Inverter/1 -> Assert/1
  Assert/1 -> Register1/1
  [mix_sync] -> Register1/2, Register2/3, Register2/1
  Register2/1 -> Logical2/1
  Logical2/1 -> Register2/2, edge_detect2/1(in)
  Logical1/1 -> Logical2/2, edge_detect3/1(in)
  [mix_dvalid] -> Logical1/2, Register1/3
  Delay9/1 -> [ddc_dvalid]
  From16/1 -> pipeline42/1(d), pipeline43/1(d)
  From13/1 -> pipeline41/1(d), pipeline44/1(d)
  pipeline44/1(q) -> snap_mix/6(trig)
  pipeline43/1(q) -> snap_mix/5(we)
  pipeline42/1(q) -> snap_mix/4(in_dv)
  pipeline41/1(q) -> snap_mix/3(in_sync)
  pipeline38/1(q) -> Reinterpret4/1
  pipeline37/1(q) -> snap_mix/1(in_mix0)
  Reinterpret4/1 -> snap_mix/2(in_mix7)
  bus_expand7/1(msb_out16) -> Gateway Out32/1, pipeline36/1(d), pipeline37/1(d)
  bus_expand7/16(lsb_out1) -> pipeline35/1(d), pipeline38/1(d)
  Reinterpret3/1 -> snap_mult/7(in_mix7)
  pipeline36/1(q) -> snap_mult/6(in_mix0)
  pipeline35/1(q) -> Reinterpret3/1
  Reinterpret1/1 -> snap_mult/4(in_bin7)
  Reinterpret/1 -> snap_mult/2(in_ain7)
  pipeline16/1(q) -> snap_mult/11(in_mix_dv)
  pipeline5/1(q) -> snap_mult/10(in_mix_sync)
  From6/1 -> pipeline34/1(d)
  From5/1 -> pipeline33/1(d)
  pipeline34/1(q) -> snap_mult/13(in_dec_dv)
  pipeline33/1(q) -> snap_mult/12(in_dec_sync)
  bus_expand3/1(msb_out16) -> pipeline3/1(d)
  bus_expand1/15(out2) -> pipeline12/1(d)
  bus_expand1/1(msb_out16) -> Gateway Out25/1, pipeline13/1(d)
  pipeline12/1(q) -> Reinterpret1/1
  pipeline14/1(q) -> Reinterpret/1
  bus_expand2/15(out2) -> pipeline14/1(d)
  bus_expand2/1(msb_out16) -> Gateway Out24/1, pipeline15/1(d)
  pipeline17/1(q) -> snap_mult/8(in_sync_in)
  pipeline4/1(q) -> snap_mult/9(in_dv_in)
  pipeline15/1(q) -> snap_mult/1(in_ain0)
  pipeline13/1(q) -> snap_mult/3(in_bin0)
  pipeline3/1(q) -> snap_mult/5(in_mout0)
  pipeline2/1(q) -> snap_mult/15(trig)
  pipeline11/1(q) -> snap_mult/14(we)
  sync/1 -> pipeline17/1(d), pipeline2/1(d), Concat/1
  dvalid/1 -> Gateway Out26/1, Concat/2, pipeline4/1(d), pipeline11/1(d)
  osc0/1 -> bus_mult/2(b), bus_expand1/1(bus_in)
  pol0_data/1 -> bus_mult/1(a), bus_expand2/1(bus_in)
  bus_expand/1(msb_out2) -> pipeline5/1(d), Gateway Out1/1, [mix_sync]
  Reinterpret2/1 -> snap_ddc/2(in_fir_re_u)
  pipeline1/1(q) -> Reinterpret2/1
  dec_fir_async0/3(dv_out) -> Delay9/1, pipeline30/1(d)
  dec_fir_async0/1(sync_out) -> [ddc_sync], pipeline29/1(d)
  Gateway Out32/1 -> To Workspace32/1
  Gateway Out1/1 -> To Workspace1/1
  Gateway Out2/1 -> To Workspace2/1
  bus_expand/2(lsb_out1) -> pipeline16/1(d), Gateway Out2/1, [mix_dvalid], Gateway Out16/1
  Gateway Out3/1 -> To Workspace3/1
  Gateway Out4/1 -> To Workspace4/1
  Gateway Out5/1 -> To Workspace5/1
  Constant2/1 -> Reinterpret5/1
  Constant3/1 -> Reinterpret6/1
  Mux/1 -> Concat3/1
  [dec_sync] -> Mux/1
  Reinterpret6/1 -> Mux/3
  Reinterpret5/1 -> Mux/2, Concat3/4, Concat3/5, Concat3/6, Concat3/7, Concat3/8, Concat3/9, Concat3/10, Concat3/11, Concat3/12, Concat3/13, Concat3/14, Concat3/15, Concat3/16, Concat3/3, Concat3/2
  Gateway Out6/1 -> To Workspace6/1
  dec_fir_async0/2(dout) -> Delay4/1
  [mix_80_1] -> [dec_d80_1]
  Delay4/1 -> bus_expand4/1(bus_in), [ddc_pol1], [ddc_pol0]
  Mux1/1 -> [dec_d80_0]
  Concat3/1 -> Mux1/2
  [mix_80_0] -> Mux1/3
  [ddc_input_sel] -> Mux2/1, Mux3/1, Mux1/1
  Mux3/1 -> pipeline31/1(d), pipeline26/1(d), dec_fir_async0/2(dv_in), Gateway Out5/1
  Mux2/1 -> pipeline32/1(d), pipeline24/1(d), Gateway Out6/1, dec_fir_async0/1(sync_in)
  [dec_sync] -> Mux2/3, Delay1/1
  [dec_dv] -> Mux3/3, Delay6/1
  Delay6/1 -> Mux3/2
  Delay1/1 -> Mux2/2
  Gateway Out7/1 -> To Workspace7/1
  bus_expand8/1(msb_out16) -> Gateway Out7/1
  Gateway Out8/1 -> To Workspace8/1
  Gateway Out9/1 -> To Workspace9/1
  bus_expand8/3(out14) -> Gateway Out9/1
  Gateway Out10/1 -> To Workspace10/1
  bus_expand8/5(out12) -> Gateway Out10/1
  Gateway Out11/1 -> To Workspace11/1
  bus_expand8/7(out10) -> Gateway Out11/1
  Gateway Out12/1 -> To Workspace12/1
  bus_expand8/9(out8) -> Gateway Out12/1
  Gateway Out13/1 -> To Workspace13/1
  bus_expand8/11(out6) -> Gateway Out13/1
  Gateway Out14/1 -> To Workspace14/1
  bus_expand8/13(out4) -> Gateway Out14/1
  Gateway Out15/1 -> To Workspace15/1
  bus_expand8/15(out2) -> Gateway Out15/1
  bus_expand8/2(out15) -> Gateway Out8/1
  Gateway Out17/1 -> To Workspace17/1
  bus_expand8/4(out13) -> Gateway Out17/1
  Gateway Out18/1 -> To Workspace18/1
  bus_expand8/6(out11) -> Gateway Out18/1
  Gateway Out19/1 -> To Workspace19/1
  bus_expand8/8(out9) -> Gateway Out19/1
  Gateway Out20/1 -> To Workspace20/1
  bus_expand8/10(out7) -> Gateway Out20/1
  Gateway Out21/1 -> To Workspace21/1
  bus_expand8/12(out5) -> Gateway Out21/1
  Gateway Out22/1 -> To Workspace22/1
  bus_expand8/14(out3) -> Gateway Out22/1
  Gateway Out23/1 -> To Workspace23/1
  bus_expand8/16(lsb_out1) -> Gateway Out23/1
  Gateway Out16/1 -> To Workspace16/1
  bus_mult1/1(a*b) -> [mix_80_1]
  bus_mult/1(a*b) -> bus_expand7/1(bus_in), [mix_80_0], bus_expand8/1(bus_in), bus_expand3/1(bus_in)
  Gateway Out24/1 -> To Workspace24/1
  Gateway Out25/1 -> To Workspace25/1
  Gateway Out26/1 -> To Workspace26/1
  Concat/1 -> bus_mult/3(misci)
